simulink model "ofdm_txrx_supermimo"  (Simulink 7.1)
settings: Solver=VariableStepDiscrete  SolverMode=SingleTasking  StartTime=0.0  StopTime=simOnly_simLength  RelTol=1e-3
PostLoadFcn:
  | ofdm_rx_supermimo_init
InitFcn:
  | ofdm_rx_supermimo_init
StartFcn:
  | ofdm_rx_supermimo_init
values from neighbouring files (symbols this model uses):
  Action0_Config = 0* 31*2^18 + 0*2^24 + PHY_AUTORESPONSE_REQ_GOODHDR + PHY_AUTORESPONSE_REQ_GOODPKT + PHY_AUTORESPONSE_REQ_FLAGA    [ofdm_tx_supermimo_init.m]
  Action1_Config = 0* 62*2^18 + PHY_AUTORESPONSE_REQ_GOODHDR + PHY_AUTORESPONSE_REQ_GOODPKT    [ofdm_tx_supermimo_init.m]
  Action2_Config = 0    [ofdm_tx_supermimo_init.m]
  Action3_Config = 0    [ofdm_tx_supermimo_init.m]
  Action4_Config = 0    [ofdm_tx_supermimo_init.m]
  Action5_Config = 0    [ofdm_tx_supermimo_init.m]
  ActionID_AFTransmit = 31    [ofdm_tx_supermimo_init.m]
  ActionID_Disabled = 0    [ofdm_tx_supermimo_init.m]
  ActionID_SetFlagA = 62    [ofdm_tx_supermimo_init.m]
  ActionID_SetFlagB = 61    [ofdm_tx_supermimo_init.m]
  BER_RAM_init_size = 2048    [ofdm_tx_supermimo_init.m]
  CPLength = 16    [ofdm_tx_supermimo_init.m]
  longCorr_coef_i = [-4*real(LTS) 0]    [ofdm_rx_supermimo_init.m]
  longCorr_coef_q = [4*imag(LTS) 0]    [ofdm_rx_supermimo_init.m]
  Match0_Config = 0    [ofdm_tx_supermimo_init.m]
  Match1_Config = 0    [ofdm_tx_supermimo_init.m]
  Match2_Config = 0    [ofdm_tx_supermimo_init.m]
  Match3_Config = 0    [ofdm_tx_supermimo_init.m]
  Match4_Config = 0    [ofdm_tx_supermimo_init.m]
  Match5_Config = 0    [ofdm_tx_supermimo_init.m]
  Match6_Config = 0    [ofdm_tx_supermimo_init.m]
  Match7_Config = 0    [ofdm_tx_supermimo_init.m]
  max_CP_length = 16    [ofdm_tx_supermimo_init.m]
  max_num_baseRateSymbols = 31    [ofdm_tx_supermimo_init.m]
  max_num_subcarriers = 64    [ofdm_tx_supermimo_init.m]
  max_num_trainingSymbols = 15    [ofdm_tx_supermimo_init.m]
  max_numBytes = 16384    [ofdm_tx_supermimo_init.m]
  max_OFDM_symbols = 511    [ofdm_tx_supermimo_init.m]
  modConstellation_qam16 = .75*[1 3 -1 -3]./3    [ofdm_tx_supermimo_init.m]
  modConstellation_qam64 = 0.875*[3 1 5 7 -3 -1 -5 -7]./7    [ofdm_tx_supermimo_init.m]
  modConstellation_qpsk = [1 -1]./sqrt(2)    [ofdm_tx_supermimo_init.m]
  numBaseRateSymbols = ceil(numHeaderBytes / numBytes_BaseRateOFDMSymbol)    [ofdm_tx_supermimo_init.m]
  numSubcarriers = 64    [ofdm_tx_supermimo_init.m]
  numTrainingSymbols = 2    [ofdm_tx_supermimo_init.m]
  pilot_indicies = 7 + ( (64-21) * 2^8) + ( (64-7) * 2^16) + (21 * 2^24)    (= 356068103)    [ofdm_tx_supermimo_init.m]
  pilotValues = hex2dec('A57D') + (2^16 * hex2dec('5A82'))    [ofdm_tx_supermimo_init.m]
  pkt_crossCorr_thresh = 0.7    [ofdm_rx_supermimo_init.m]
  pkt_energy_thresh = 0    [ofdm_rx_supermimo_init.m]
  pktByteNums = numHeaderBytes +  				byteIndex_numPayloadBytes(1)*2^8 +  				byteIndex_numPayloadBytes(2)*2^16 +  				byteIndex_simpleDynModMasks * 2^24    [ofdm_rx_supermimo_init.m]
  PktDet_Delay = 58+32 +      Symbol_Timing_Offset*2^7 +      0*2^16 +      (117* 2^24)    (= 1962935642)    [ofdm_rx_supermimo_init.m]
  pktTiming_count_payloadStart = pktTiming_count_lsStart + pktTiming_ls_num*pktTiming_ls_length    [ofdm_rx_supermimo_init.m]
  preamble = preamble_scale*[shortsyms_10 longsyms_2]    [ofdm_tx_supermimo_init.m]
  preamble_ROM = preamble_I_ROM + preamble_Q_ROM*2^16    [ofdm_tx_supermimo_init.m]
  RAM_init_size = max_numBytes    (= 16384)    [ofdm_tx_supermimo_init.m]
  reg_AF_Tx_Blanking = (320+80) + ( (320+80+79)*2^16)    (= 31392144)    [ofdm_rx_supermimo_init.m]
  reg_AF_Tx_Scaling = 2944    [ofdm_rx_supermimo_init.m]
  reg_Interrupt_PktBuf_Control = reg_InterruptControl +  	1 * 2^16 +  	0 * 2^24    (= 65656)    [ofdm_rx_supermimo_init.m]
  reg_PilotCalcParams = (4 * 2^16) + (minPilotChanMag)    (= 262184.96)    [ofdm_rx_supermimo_init.m]
  regRx_chanEst_minMag = ((0.5)*2^15) + ((0.5 * 2^15)*2^16)    (= 1073758208)    [ofdm_rx_supermimo_init.m]
  regRx_coarseCalcCorrection = round((1.3e-3 - 1.2982e-3)*2^32)    [ofdm_rx_supermimo_init.m]
  regRx_pilotCalcCorrection = round(1.00115 * 2^31)    [ofdm_rx_supermimo_init.m]
  regRx_preCFOoptions = 0 * 2^0 +  	1 * 2^1 +  	0    (= 2)    [ofdm_rx_supermimo_init.m]
  rx_controlBits = 1 * 2  		 + 1 * 4  		 + 0 * 8  		 + 1 * 16 * rx_SISO_Mode  		 + 1 * 32  		 + 1 * 64  		 + 1 * 128  		 + 1 * 256  		 + 1 * 512  		 + 1 * 1024  		 + 1 * 4096  		 + 0 * 16384  		 + 0 * 32768  		 + 1 * 65536  		 + 0 * 131072  		 + 0 * 262144  		 + 1 * 524288  		 + 1 * 1048576 * tx_Alamouti_Mode  		 + 0 * 2097152           + 1 * 33554432  		 + 0 * 4194304  		 + 0 * 8388608  		 + 0 * 16777216  		 + 1 *  ...(+49 chars)    [ofdm_rx_supermimo_init.m]
  Rx_PktDet_LongCorr_Params = LongCorrCounterLoad + 0*2^8 + 0*2^16 + 255*2^24    (= 4278190325)    [ofdm_rx_supermimo_init.m]
  Rx_PktDet_LongCorrThresholds = 8000    [ofdm_rx_supermimo_init.m]
  rx_postEq_scaling = round(rxScaling*2^11) * (1 + 2^16)    [ofdm_rx_supermimo_init.m]
  RxDataScrambling_Seq = [40 198 78 63 82 173 102 245 48 111 172 115 147 230 216 93 72 65 62 2 205 242 122 90 128 83 105 97 73 10 5 252]    [ofdm_tx_supermimo_init.m]
  RxReg_FixedPktLen = 0    [ofdm_rx_supermimo_init.m]
  shortSymbol_time = shortSymbol_time(1:16).'    [ofdm_tx_supermimo_init.m]
  simOnly_numSamples = length(preamble)+( (numSubcarriers+CPLength)*(numTrainingSymbols + numBaseRateSymbols + numFullRateSymbols/2) )    [ofdm_tx_supermimo_init.m]
  train = [train train]    [ofdm_tx_supermimo_init.m]
  tx_controlBits = (antB_preambleShift * 2^4) + (2^1 * tx_Alamouti_Mode) +  	(2^0 * tx_SISO_Mode) + 4*0 + 8*1 + 0*256 + 512*0 + 1024*0 +  	0*hex2dec('1000') + 0*hex2dec('2000') + 0*hex2dec('4000') +  	1*hex2dec('8000') + 1*hex2dec('10000') + hex2dec('20000') +  	16*hex2dec('100000')    [ofdm_tx_supermimo_init.m]
  tx_delays = (50*2^0) + (0*2^8) + (30*2^12)    (= 122930)    [ofdm_tx_supermimo_init.m]
  tx_scaling = (3072) + (12288 * 2^16)    (= 805309440)    [ofdm_tx_supermimo_init.m]
  TxDataScrambling_Seq = [40 198 78 63 82 173 102 245 48 111 172 115 147 230 216 93 72 65 62 2 205 242 122 90 128 83 105 97 73 10 5 252]    [ofdm_tx_supermimo_init.m]
  txfilt_firpm = 0.95*txfilt_firpm./(max(txfilt_firpm))    [ofdm_tx_supermimo_init.m]
  txfilt_intfilt = [0 intfilt(4,4,.8)]    [ofdm_tx_supermimo_init.m]
  txReg_symbolCounts = (2^8 *numBaseRateSymbols ) + numTrainingSymbols    [ofdm_tx_supermimo_init.m]
  TxRx_FFTScaling = (3 * 2^0) +  	(2 * 2^2) +  	(1 * 2^4) +  	(1 * 2^6) +  	(1 * 2^8) +  	(0 * 2^10)    (= 347)    [ofdm_tx_supermimo_init.m]
undefined symbols (not defined in the file or in its folder): AntA, AntB, BaseRate, Down, gcbh, numBuffs, numBytes, Up, xlBlockLoadCallback, xlGetSimulinkPeriod, xlipgetpartsetting
libraries: xbsIndex_r4 x6035, simulink x4, dspsigops x4, commchan3 x2, dspsrcs4 x2, commrflib2 x2
(part 25 of 61)

== system ofdm_txrx_supermimo/OFDM Rx MIMO/Packet_Detection/Internal PktDet (Sim Only!)/Magnitude Squared ==
  I : Inport  OutDataType=sfix(16)  OutScaling=2^0
  Q : Inport  Port=2  OutDataType=sfix(16)  OutScaling=2^0
  AddSub1 : xbsIndex_r4/AddSub  mode=Addition  use_carryin=off  use_carryout=off  en=off  latency=addLatency  precision=User Defined  arith_type=Unsigned  n_bits=prec  bin_pt=bp  quantization=Truncate  overflow=Saturate  dbl_ovrd=off  use_behavioral_HDL=on  pipelined=off  use_rpm=on  hw_selection=Fabric  block_type=addsub
      settings: xl_area=[12 22 0 22 0 0 0]  block_version=VER_STRING_GOES_HERE
  Mult1 : xbsIndex_r4/Mult  precision=User Defined  arith_type="Signed  (2's comp)"  n_bits=prec  bin_pt=bp+1  quantization=Truncate  overflow=Saturate  en=off  latency=multLatency  dbl_ovrd=off  use_behavioral_HDL=off  use_embedded=on  opt=Speed  optimum_pipeline=off  pipeline=on  use_rpm=off  placement_style=Rectangular shape  block_type=mult
      settings: infoedit=Hardware notes: To use the internal pipeline stage of the dedicated multiplier you must select 'Pipeline for maximum performance'.  xl_area=[63 105 0 106 0 4 0]  block_version=VER_STRING_GOES_HERE
  Mult4 : xbsIndex_r4/Mult  precision=User Defined  arith_type="Signed  (2's comp)"  n_bits=prec  bin_pt=bp+1  quantization=Truncate  overflow=Saturate  en=off  latency=multLatency  dbl_ovrd=off  use_behavioral_HDL=off  use_embedded=on  opt=Speed  optimum_pipeline=off  pipeline=on  use_rpm=off  placement_style=Rectangular shape  block_type=mult
      settings: infoedit=Hardware notes: To use the internal pipeline stage of the dedicated multiplier you must select 'Pipeline for maximum performance'.  xl_area=[63 105 0 106 0 4 0]  block_version=VER_STRING_GOES_HERE
  Mag : Outport  OutDataType=sfix(16)  OutScaling=2^0
  wires:
  AddSub1/1 -> Mag/1
  Mult1/1 -> AddSub1/2
  Mult4/1 -> AddSub1/1
  Q/1 -> Mult1/2, Mult1/1
  I/1 -> Mult4/2, Mult4/1

== system ofdm_txrx_supermimo/OFDM Rx MIMO/Packet_Detection/Internal PktDet (Sim Only!)/Magnitude Squared1 ==
  I : Inport  OutDataType=sfix(16)  OutScaling=2^0
  Q : Inport  Port=2  OutDataType=sfix(16)  OutScaling=2^0
  AddSub1 : xbsIndex_r4/AddSub  mode=Addition  use_carryin=off  use_carryout=off  en=off  latency=addLatency  precision=User Defined  arith_type=Unsigned  n_bits=prec  bin_pt=bp  quantization=Truncate  overflow=Saturate  dbl_ovrd=off  use_behavioral_HDL=on  pipelined=off  use_rpm=on  hw_selection=Fabric  block_type=addsub
      settings: xl_area=[9 0 0 17 0 0 0]  block_version=VER_STRING_GOES_HERE
  Mult1 : xbsIndex_r4/Mult  precision=User Defined  arith_type="Signed  (2's comp)"  n_bits=prec  bin_pt=bp+1  quantization=Truncate  overflow=Saturate  en=off  latency=multLatency  dbl_ovrd=off  use_behavioral_HDL=off  use_embedded=on  opt=Speed  optimum_pipeline=off  pipeline=on  use_rpm=off  placement_style=Rectangular shape  block_type=mult
      settings: infoedit=Hardware notes: To use the internal pipeline stage of the dedicated multiplier you must select 'Pipeline for maximum performance'.  xl_area=[9 17 0 17 0 1 0]  block_version=VER_STRING_GOES_HERE
  Mult4 : xbsIndex_r4/Mult  precision=User Defined  arith_type="Signed  (2's comp)"  n_bits=prec  bin_pt=bp+1  quantization=Truncate  overflow=Saturate  en=off  latency=multLatency  dbl_ovrd=off  use_behavioral_HDL=off  use_embedded=on  opt=Speed  optimum_pipeline=off  pipeline=on  use_rpm=off  placement_style=Rectangular shape  block_type=mult
      settings: infoedit=Hardware notes: To use the internal pipeline stage of the dedicated multiplier you must select 'Pipeline for maximum performance'.  xl_area=[9 17 0 17 0 1 0]  block_version=VER_STRING_GOES_HERE
  Mag : Outport  OutDataType=sfix(16)  OutScaling=2^0
  wires:
  I/1 -> Mult4/1, Mult4/2
  Q/1 -> Mult1/1, Mult1/2
  Mult4/1 -> AddSub1/1
  Mult1/1 -> AddSub1/2
  AddSub1/1 -> Mag/1

== system ofdm_txrx_supermimo/OFDM Rx MIMO/Packet_Detection/Internal PktDet (Sim Only!)/Sliding Window ==
  In : Inport  OutDataType=sfix(16)  OutScaling=2^0
  Accum1 : xbsIndex_r4/Accumulator  operation=Add  n_bits=22  overflow=Flag as error  scale=1  rst=off  hasbypass=off  en=off  dbl_ovrd=off  use_behavioral_HDL=on  implementation=Fabric  block_type=accum
      settings: infoedit="Adder or subtractor-based accumulator.   Output type and binary point position match the input.<P><P>Hardware notes: When \"Reinitialize with input 'b' on reset\" is selected, the accumulator is forced to run at the system rate even if the input 'b' is running at a slower rate."  xl_area=[12 23 0 22 0 0 0]  block_version=VER_STRING_GOES_HERE
  AddSub1 : xbsIndex_r4/AddSub  mode=Subtraction  use_carryin=off  use_carryout=off  en=off  latency=1  precision=Full  arith_type="Signed  (2's comp)"  n_bits=36  bin_pt=28  quantization=Truncate  overflow=Saturate  dbl_ovrd=off  use_behavioral_HDL=on  pipelined=off  use_rpm=off  hw_selection=Fabric  block_type=addsub
      settings: xl_area=[9 18 0 17 0 0 0]  block_version=VER_STRING_GOES_HERE
  Delay6 : xbsIndex_r4/Delay  en=off  latency=16  dbl_ovrd=off  reg_retiming=off  block_type=delay
      settings: infoedit=Hardware notes: A delay line is a chain, each link of which is an SRL16 followed by a flip-flop. If register retiming is enabled, the delay line is a chain of flip-flops.  xl_area=[9 17 0 17 0 0 0]  block_version=VER_STRING_GOES_HERE
  Out : Outport  OutDataType=sfix(16)  OutScaling=2^0
  wires:
  Accum1/1 -> Out/1
  In/1 -> Delay6/1, AddSub1/1
  AddSub1/1 -> Accum1/1
  Delay6/1 -> AddSub1/2

== system ofdm_txrx_supermimo/OFDM Rx MIMO/Packet_Detection/Internal PktDet (Sim Only!)/Sliding Window1 ==
  In : Inport  OutDataType=sfix(16)  OutScaling=2^0
  Accum1 : xbsIndex_r4/Accumulator  operation=Add  n_bits=22  overflow=Flag as error  scale=1  rst=off  hasbypass=off  en=off  dbl_ovrd=off  use_behavioral_HDL=on  implementation=Fabric  block_type=accum
      settings: infoedit="Adder or subtractor-based accumulator.   Output type and binary point position match the input.<P><P>Hardware notes: When \"Reinitialize with input 'b' on reset\" is selected, the accumulator is forced to run at the system rate even if the input 'b' is running at a slower rate."  xl_area=[12 23 0 22 0 0 0]  block_version=VER_STRING_GOES_HERE
  AddSub1 : xbsIndex_r4/AddSub  mode=Subtraction  use_carryin=off  use_carryout=off  en=off  latency=1  precision=Full  arith_type="Signed  (2's comp)"  n_bits=36  bin_pt=28  quantization=Truncate  overflow=Saturate  dbl_ovrd=off  use_behavioral_HDL=on  pipelined=off  use_rpm=off  hw_selection=Fabric  block_type=addsub
      settings: xl_area=[9 18 0 17 0 0 0]  block_version=VER_STRING_GOES_HERE
  Delay6 : xbsIndex_r4/Delay  en=off  latency=16  dbl_ovrd=off  reg_retiming=off  block_type=delay
      settings: infoedit=Hardware notes: A delay line is a chain, each link of which is an SRL16 followed by a flip-flop. If register retiming is enabled, the delay line is a chain of flip-flops.  xl_area=[9 17 0 17 0 0 0]  block_version=VER_STRING_GOES_HERE
  Out : Outport  OutDataType=sfix(16)  OutScaling=2^0
  wires:
  Delay6/1 -> AddSub1/2
  AddSub1/1 -> Accum1/1
  In/1 -> AddSub1/1, Delay6/1
  Accum1/1 -> Out/1

== system ofdm_txrx_supermimo/OFDM Rx MIMO/Packet_Detection/Internal PktDet (Sim Only!)/Sliding Window2 ==
  In : Inport  OutDataType=sfix(16)  OutScaling=2^0
  Accum1 : xbsIndex_r4/Accumulator  operation=Add  n_bits=22  overflow=Flag as error  scale=1  rst=off  hasbypass=off  en=off  dbl_ovrd=off  use_behavioral_HDL=on  implementation=Fabric  block_type=accum
      settings: infoedit="Adder or subtractor-based accumulator.   Output type and binary point position match the input.<P><P>Hardware notes: When \"Reinitialize with input 'b' on reset\" is selected, the accumulator is forced to run at the system rate even if the input 'b' is running at a slower rate."  xl_area=[12 23 0 22 0 0 0]  block_version=VER_STRING_GOES_HERE
  AddSub1 : xbsIndex_r4/AddSub  mode=Subtraction  use_carryin=off  use_carryout=off  en=off  latency=0  precision=Full  arith_type="Signed  (2's comp)"  n_bits=36  bin_pt=28  quantization=Truncate  overflow=Saturate  dbl_ovrd=off  use_behavioral_HDL=on  pipelined=off  use_rpm=off  hw_selection=Fabric  block_type=addsub
      settings: xl_area=[9 0 0 17 0 0 0]  block_version=VER_STRING_GOES_HERE
  Delay6 : xbsIndex_r4/Delay  en=off  latency=16  dbl_ovrd=off  reg_retiming=off  block_type=delay
      settings: infoedit=Hardware notes: A delay line is a chain, each link of which is an SRL16 followed by a flip-flop. If register retiming is enabled, the delay line is a chain of flip-flops.  xl_area=[9 17 0 17 0 0 0]  block_version=VER_STRING_GOES_HERE
  Out : Outport  OutDataType=sfix(16)  OutScaling=2^0
  wires:
  Accum1/1 -> Out/1
  In/1 -> Delay6/1, AddSub1/1
  AddSub1/1 -> Accum1/1
  Delay6/1 -> AddSub1/2

== system ofdm_txrx_supermimo/OFDM Rx MIMO/Packet_Detection/RSSI-based PktDet/Ant A Carrier Sensing ==
  Avg RSSI : Inport  OutDataType=sfix(16)  OutScaling=2^0
  Convert : xbsIndex_r4/Convert  arith_type=Unsigned  n_bits=1  bin_pt=0  quantization=Truncate  overflow=Wrap  latency=0  dbl_ovrd=off  pipeline=off  block_type=convert
      settings: block_version=8.2
  Convert1 : xbsIndex_r4/Convert  arith_type=Unsigned  n_bits=1  bin_pt=0  quantization=Truncate  overflow=Wrap  latency=0  dbl_ovrd=off  pipeline=off  block_type=convert
      settings: block_version=8.2
  Inverter : xbsIndex_r4/Inverter  en=off  latency=0  dbl_ovrd=off  block_type=inv
  Inverter1 : xbsIndex_r4/Inverter  en=off  latency=0  dbl_ovrd=off  block_type=inv
      settings: block_version=8.2
  Logical6 : xbsIndex_r4/Logical  logical_function=AND  inputs=2  en=off  latency=0  precision=Full  arith_type=Unsigned  n_bits=16  bin_pt=0  align_bp=on  dbl_ovrd=off  block_type=logical
      settings: block_version=8.2
  Logical7 : xbsIndex_r4/Logical  logical_function=OR  inputs=2  en=off  latency=0  precision=Full  arith_type=Unsigned  n_bits=16  bin_pt=0  align_bp=on  dbl_ovrd=off  block_type=logical
      settings: block_version=8.2
  Relational1 : xbsIndex_r4/Relational  mode=a>b  en=off  latency=0  dbl_ovrd=off  block_type=relational
      settings: block_version=VER_STRING_GOES_HERE
  busy : Outport  OutDataType=sfix(16)  OutScaling=2^0
  idle : Outport  Port=2  OutDataType=sfix(16)  OutScaling=2^0
  wires:
  Avg RSSI/1 -> Relational1/1
  Relational1/1 -> Convert/1
  Convert/1 -> Inverter/1, Logical6/2
  [regPktDet_carrierSenseThresh] -> Relational1/2
  Inverter/1 -> Logical7/1
  [regPktDet_pktDetMask_A] -> Convert1/1
  Inverter1/1 -> Logical7/2
  Logical6/1 -> busy/1
  Logical7/1 -> idle/1
  Convert1/1 -> Inverter1/1, Logical6/1

== system ofdm_txrx_supermimo/OFDM Rx MIMO/Packet_Detection/RSSI-based PktDet/Ant B Carrier Sensing ==
  Avg RSSI : Inport  OutDataType=sfix(16)  OutScaling=2^0
  Convert1 : xbsIndex_r4/Convert  arith_type=Unsigned  n_bits=1  bin_pt=0  quantization=Truncate  overflow=Wrap  latency=0  dbl_ovrd=off  pipeline=off  block_type=convert
      settings: block_version=8.2
  Convert2 : xbsIndex_r4/Convert  arith_type=Unsigned  n_bits=1  bin_pt=0  quantization=Truncate  overflow=Wrap  latency=0  dbl_ovrd=off  pipeline=off  block_type=convert
      settings: block_version=8.2
  Inverter : xbsIndex_r4/Inverter  en=off  latency=0  dbl_ovrd=off  block_type=inv
  Inverter1 : xbsIndex_r4/Inverter  en=off  latency=0  dbl_ovrd=off  block_type=inv
      settings: block_version=8.2
  Logical6 : xbsIndex_r4/Logical  logical_function=AND  inputs=2  en=off  latency=0  precision=Full  arith_type=Unsigned  n_bits=16  bin_pt=0  align_bp=on  dbl_ovrd=off  block_type=logical
      settings: block_version=8.2
  Logical7 : xbsIndex_r4/Logical  logical_function=OR  inputs=2  en=off  latency=0  precision=Full  arith_type=Unsigned  n_bits=16  bin_pt=0  align_bp=on  dbl_ovrd=off  block_type=logical
      settings: block_version=8.2
  Relational1 : xbsIndex_r4/Relational  mode=a>b  en=off  latency=0  dbl_ovrd=off  block_type=relational
      settings: block_version=VER_STRING_GOES_HERE
  busy : Outport  OutDataType=sfix(16)  OutScaling=2^0
  idle : Outport  Port=2  OutDataType=sfix(16)  OutScaling=2^0
  wires:
  Logical7/1 -> idle/1
  Logical6/1 -> busy/1
  Inverter1/1 -> Logical7/2
  [regPktDet_pktDetMask_B] -> Convert2/1
  Inverter/1 -> Logical7/1
  [regPktDet_carrierSenseThresh] -> Relational1/2
  Convert1/1 -> Logical6/2, Inverter/1
  Relational1/1 -> Convert1/1
  Avg RSSI/1 -> Relational1/1
  Convert2/1 -> Logical6/1, Inverter1/1

== system ofdm_txrx_supermimo/OFDM Rx MIMO/Packet_Detection/RSSI-based PktDet/AntA Detector ==
  RSSI : Inport  OutDataType=sfix(16)  OutScaling=2^0
  Reset : Inport  Port=2  OutDataType=sfix(16)  OutScaling=2^0
  Detection Decision : Subsystem
  Gateway Out : xbsIndex_r4/Gateway Out  hdl_port=off  timing_constraint=None  locs_specified=off  LOCs={}  block_type=gatewayout
      settings: infoedit="Gateway out block.  Converts Xilinx fixed point inputs into ouputs of type Simulink integer, double, or fixed point.<P><P>Hardware notes:  In hardware these blocks become top level output ports or are discarded, depending on how they are configured."  block_version=8.2
  Gateway Out1 : xbsIndex_r4/Gateway Out  hdl_port=off  timing_constraint=None  locs_specified=off  LOCs={}  block_type=gatewayout
      settings: infoedit="Gateway out block.  Converts Xilinx fixed point inputs into ouputs of type Simulink integer, double, or fixed point.<P><P>Hardware notes:  In hardware these blocks become top level output ports or are discarded, depending on how they are configured."  block_version=8.2
  Gateway Out2 : xbsIndex_r4/Gateway Out  hdl_port=off  timing_constraint=None  locs_specified=off  LOCs={}  block_type=gatewayout
      settings: infoedit="Gateway out block.  Converts Xilinx fixed point inputs into ouputs of type Simulink integer, double, or fixed point.<P><P>Hardware notes:  In hardware these blocks become top level output ports or are discarded, depending on how they are configured."  block_version=8.2
  Pkt Det Decision : Scope  NumInputPorts=3
      settings: YMin=-1~-1~-1  YMax=1~1~1
  RF Gain Adj : Subsystem
  Running Sum : Subsystem
  pktdet : Outport  OutDataType=sfix(16)  OutScaling=2^0
  avgRSSI : Outport  Port=2  OutDataType=sfix(16)  OutScaling=2^0
  tag scope: regPktDet_pktDetected_AntA=global
  wires:
  Running Sum/1(avg) -> avgRSSI/1, Gateway Out1/1, Detection Decision/1(Avg RSSI)
  [regPktDet_MasterReset] -> Running Sum/2(reset)
  [regPktDet_RSSIavgLength] -> Running Sum/3(avglen)
  RF Gain Adj/1(" RSSI") -> Running Sum/1(RSSI), Gateway Out/1
  Gateway Out/1 -> Pkt Det Decision/1  "RAWRSSI"
  Gateway Out1/1 -> Pkt Det Decision/2  "AVGRSSI"
  Gateway Out2/1 -> Pkt Det Decision/3  "PKTDET"
  Reset/1 -> Detection Decision/2(Reset)
  Detection Decision/1(Pkt Det) -> pktdet/1, Gateway Out2/1, [regPktDet_pktDetected_AntA]
  RSSI/1 -> RF Gain Adj/1(RSSI)

== system ofdm_txrx_supermimo/OFDM Rx MIMO/Packet_Detection/RSSI-based PktDet/AntB Detector ==
  RSSI : Inport  OutDataType=sfix(16)  OutScaling=2^0
  Reset : Inport  Port=2  OutDataType=sfix(16)  OutScaling=2^0
  Detection Decision : Subsystem
  Gateway Out : xbsIndex_r4/Gateway Out  hdl_port=off  timing_constraint=None  locs_specified=off  LOCs={}  block_type=gatewayout
      settings: infoedit="Gateway out block.  Converts Xilinx fixed point inputs into ouputs of type Simulink integer, double, or fixed point.<P><P>Hardware notes:  In hardware these blocks become top level output ports or are discarded, depending on how they are configured."  block_version=8.2
  Gateway Out1 : xbsIndex_r4/Gateway Out  hdl_port=off  timing_constraint=None  locs_specified=off  LOCs={}  block_type=gatewayout
      settings: infoedit="Gateway out block.  Converts Xilinx fixed point inputs into ouputs of type Simulink integer, double, or fixed point.<P><P>Hardware notes:  In hardware these blocks become top level output ports or are discarded, depending on how they are configured."  block_version=8.2
  Gateway Out2 : xbsIndex_r4/Gateway Out  hdl_port=off  timing_constraint=None  locs_specified=off  LOCs={}  block_type=gatewayout
      settings: infoedit="Gateway out block.  Converts Xilinx fixed point inputs into ouputs of type Simulink integer, double, or fixed point.<P><P>Hardware notes:  In hardware these blocks become top level output ports or are discarded, depending on how they are configured."  block_version=8.2
  Pkt Det Decision : Scope  NumInputPorts=3
      settings: YMin=-1~-1~-1  YMax=1~1~1
  RF Gain Adj : Subsystem
  Running Sum : Subsystem
  pktdet : Outport  OutDataType=sfix(16)  OutScaling=2^0
  avgRSSI : Outport  Port=2  OutDataType=sfix(16)  OutScaling=2^0
  tag scope: regPktDet_pktDetected_AntB=global
  wires:
  Detection Decision/1(Pkt Det) -> Gateway Out2/1, pktdet/1, [regPktDet_pktDetected_AntB]
  Reset/1 -> Detection Decision/2(Reset)
  Gateway Out2/1 -> Pkt Det Decision/3  "PKTDET"
  Gateway Out1/1 -> Pkt Det Decision/2  "AVGRSSI"
  Gateway Out/1 -> Pkt Det Decision/1  "RAWRSSI"
  RF Gain Adj/1(" RSSI") -> Gateway Out/1, Running Sum/1(RSSI)
  [regPktDet_RSSIavgLength] -> Running Sum/3(avglen)
  [regPktDet_MasterReset] -> Running Sum/2(reset)
  Running Sum/1(avg) -> Gateway Out1/1, avgRSSI/1, Detection Decision/1(Avg RSSI)
  RSSI/1 -> RF Gain Adj/1(RSSI)

== system ofdm_txrx_supermimo/OFDM Rx MIMO/Packet_Detection/RSSI-based PktDet/DIFS Counter ==
  Busy : Inport
  Idle : Inport  Port=2
  Convert1 : xbsIndex_r4/Convert  arith_type=Unsigned  n_bits=1  bin_pt=0  quantization=Truncate  overflow=Wrap  latency=0  dbl_ovrd=off  pipeline=off  block_type=convert
      settings: block_version=VER_STRING_GOES_HERE
  Convert2 : xbsIndex_r4/Convert  arith_type=Boolean  n_bits=1  bin_pt=0  quantization=Truncate  overflow=Wrap  latency=0  dbl_ovrd=off  pipeline=off  block_type=convert
      settings: block_version=VER_STRING_GOES_HERE
  Convert3 : xbsIndex_r4/Convert  arith_type=Boolean  n_bits=1  bin_pt=0  quantization=Truncate  overflow=Wrap  latency=0  dbl_ovrd=off  pipeline=off  block_type=convert
      settings: block_version=VER_STRING_GOES_HERE
  Counter1 : xbsIndex_r4/Counter  cnt_type=Free Running  cnt_to=Inf  operation=Up  start_count=0  cnt_by_val=1  arith_type=Unsigned  n_bits=10  bin_pt=0  load_pin=off  rst=on  en=on  explicit_period=off  period=4  dbl_ovrd=off  use_behavioral_HDL=off  use_rpm=off  implementation=Fabric  block_type=counter
  Inverter1 : xbsIndex_r4/Inverter  en=off  latency=0  dbl_ovrd=off  block_type=inv
      settings: xl_area=[0 0 0 0 0 0 0]  block_version=8.2
  Logical1 : xbsIndex_r4/Logical  logical_function=OR  inputs=2  en=off  latency=0  precision=Full  arith_type=Unsigned  n_bits=16  bin_pt=0  align_bp=on  dbl_ovrd=off  block_type=logical
  Relational3 : xbsIndex_r4/Relational  mode=a>b  en=off  latency=0  dbl_ovrd=off  block_type=relational
      settings: block_version=VER_STRING_GOES_HERE
  S-R Latch : Subsystem
  Idle for DIFS : Outport
  tag scope: regPktDet_idleCounter=global
  wires:
  Convert1/1 -> Idle for DIFS/1
  Idle/1 -> Convert2/1
  S-R Latch/1(Q) -> Convert1/1
  Relational3/1 -> S-R Latch/2(S)
  [regPktDet_DIFSperiod] -> Relational3/2
  Counter1/1 -> Relational3/1, [regPktDet_idleCounter]
  Inverter1/1 -> Logical1/2
  Logical1/1 -> Counter1/1
  Busy/1 -> Convert3/1
  Convert2/1 -> Counter1/2, Inverter1/1
  Convert3/1 -> S-R Latch/1(R), Logical1/1

== system ofdm_txrx_supermimo/OFDM Rx MIMO/Packet_Detection/RSSI-based PktDet/Ext PktDet ==
  Constant2 : Constant  Value=0  OutDataType=sfix(16)  OutScaling=2^0
  Convert5 : xbsIndex_r4/Convert  arith_type=Boolean  n_bits=1  bin_pt=0  quantization=Truncate  overflow=Flag as error  latency=0  dbl_ovrd=off  pipeline=off  block_type=convert
      settings: block_version=10.1.3
  Down Sample1 : xbsIndex_r4/Down Sample  sample_ratio=4  sample_phase="Last Value of Frame  (most efficient)"  en=off  latency=1  dbl_ovrd=off  block_type=dsamp
      settings: block_version=VER_STRING_GOES_HERE
  Ext_PktDet : xbsIndex_r4/Gateway In  arith_type=Unsigned  n_bits=1  bin_pt=0  quantization="Round  (unbiased: +/- Inf)"  overflow=Saturate  period=1  dbl_ovrd=off  timing_constraint=None  locs_specified=off  LOCs={}  block_type=gatewayin
      settings: infoedit="Gateway in block.  Converts inputs of type Simulink integer, double and fixed point to  Xilinx fixed point type.<P><P>Hardware notes:  In hardware these blocks become top level input ports."  block_version=VER_STRING_GOES_HERE
  Logical2 : xbsIndex_r4/Logical  logical_function=AND  inputs=2  en=off  latency=0  precision=Full  arith_type=Unsigned  n_bits=16  bin_pt=0  align_bp=on  dbl_ovrd=off  block_type=logical
      settings: block_version=8.2
  Det : Outport
  wires:
  Ext_PktDet/1 -> Down Sample1/1
  Constant2/1 -> Ext_PktDet/1
  [regPktDet_EnableExtDet] -> Logical2/2
  Logical2/1 -> Det/1
  Convert5/1 -> Logical2/1
  Down Sample1/1 -> Convert5/1

== system ofdm_txrx_supermimo/OFDM Rx MIMO/Packet_Detection/RSSI-based PktDet/MIMO Logic ==
  Det A : Inport  OutDataType=sfix(16)  OutScaling=2^0
  Det B : Inport  Port=2  OutDataType=sfix(16)  OutScaling=2^0
  Convert{1..4,7} (5 alike) : xbsIndex_r4/Convert  arith_type=Unsigned  n_bits=1  bin_pt=0  quantization=Truncate  overflow=Flag as error  latency=0  dbl_ovrd=off  pipeline=off  block_type=convert
      settings: block_version=10.1.3
  Logical : xbsIndex_r4/Logical  logical_function=AND  inputs=2  en=off  latency=0  precision=Full  arith_type=Unsigned  n_bits=16  bin_pt=0  align_bp=on  dbl_ovrd=off  block_type=logical
  Logical1 : xbsIndex_r4/Logical  logical_function=AND  inputs=2  en=off  latency=0  precision=Full  arith_type=Unsigned  n_bits=16  bin_pt=0  align_bp=on  dbl_ovrd=off  block_type=logical
  Logical2 : xbsIndex_r4/Logical  logical_function=OR  inputs=2  en=off  latency=0  precision=Full  arith_type=Unsigned  n_bits=16  bin_pt=0  align_bp=on  dbl_ovrd=off  block_type=logical
  Logical3 : xbsIndex_r4/Logical  logical_function=AND  inputs=2  en=off  latency=0  precision=Full  arith_type=Unsigned  n_bits=16  bin_pt=0  align_bp=on  dbl_ovrd=off  block_type=logical
  Mux : xbsIndex_r4/Mux  inputs=2  en=off  latency=0  precision=Full  arith_type=Unsigned  n_bits=16  bin_pt=14  quantization=Truncate  overflow=Wrap  dbl_ovrd=off  block_type=mux
  PktDet : Outport  OutDataType=sfix(16)  OutScaling=2^0
  wires:
  [regPktDet_pktDetMode] -> Convert4/1
  [regPktDet_pktDetMask_A] -> Convert2/1
  [regPktDet_pktDetMask_B] -> Convert3/1
  Det A/1 -> Convert7/1
  Det B/1 -> Convert1/1
  Convert7/1 -> Logical/1
  Convert2/1 -> Logical/2
  Convert1/1 -> Logical1/1
  Convert3/1 -> Logical1/2
  Logical/1 -> Logical2/1, Logical3/1
  Logical1/1 -> Logical2/2, Logical3/2
  Logical2/1 -> Mux/3
  Convert4/1 -> Mux/1
  Logical3/1 -> Mux/2
  Mux/1 -> PktDet/1

== system ofdm_txrx_supermimo/OFDM Rx MIMO/Packet_Detection/RSSI-based PktDet/Mid-Packet RSSI Register ==
  AntB : Inport
  AntA : Inport  Port=2
  Payload : Inport  Port=3  OutDataType=sfix(16)  OutScaling=2^0
  Concat1 : xbsIndex_r4/Concat  num_inputs=2  dbl_ovrd=off  block_type=concat
      settings: block_version=10.1.3
  Convert4 : xbsIndex_r4/Convert  arith_type=Boolean  n_bits=1  bin_pt=0  quantization=Truncate  overflow=Wrap  latency=0  dbl_ovrd=off  pipeline=off  block_type=convert
      settings: block_version=10.1.3
  Convert5 : xbsIndex_r4/Convert  arith_type=Unsigned  n_bits=16  bin_pt=0  quantization=Truncate  overflow=Wrap  latency=0  dbl_ovrd=off  pipeline=off  block_type=convert
      settings: block_version=10.1.3
  Convert6 : xbsIndex_r4/Convert  arith_type=Unsigned  n_bits=16  bin_pt=0  quantization=Truncate  overflow=Wrap  latency=0  dbl_ovrd=off  pipeline=off  block_type=convert
      settings: block_version=10.1.3
  Terminator : Terminator
  To Register2 : xbsIndex_r4/To Register  shared_memory_name='midPacketRSSI'  init=0  ownership=Locally owned and initialized  explicit_data_type=on  arith_type=Unsigned  n_bits=32  bin_pt=0  dbl_ovrd=off  block_type=toreg
      settings: AttributesFormatString=<< %<shared_memory_name> >>  infoedit="Register block that writes data to a shared memory register.  Delay of one sample period."  block_version=10.1.3
  posedge1 : Subsystem
  wires:
  Payload/1 -> posedge1/1(In)
  posedge1/1(Out) -> Convert4/1
  Concat1/1 -> To Register2/1
  To Register2/1 -> Terminator/1
  Convert4/1 -> To Register2/2
  Convert5/1 -> Concat1/1
  Convert6/1 -> Concat1/2
  AntB/1 -> Convert5/1
  AntA/1 -> Convert6/1

== system ofdm_txrx_supermimo/OFDM Rx MIMO/Packet_Detection/RSSI-based PktDet/Posedge ==
  D : Inport  OutDataType=sfix(16)  OutScaling=2^0
  Delay : xbsIndex_r4/Delay  en=off  latency=1  dbl_ovrd=off  reg_retiming=off  block_type=delay
      settings: infoedit=Hardware notes: A delay line is a chain, each link of which is an SRL16 followed by a flip-flop. If register retiming is enabled, the delay line is a chain of flip-flops.  xl_area=[1 1 0 0 0 0 0]  block_version=VER_STRING_GOES_HERE
  Inverter : xbsIndex_r4/Inverter  en=off  latency=0  dbl_ovrd=off  block_type=inv
      settings: xl_area=[0 0 0 0 0 0 0]  block_version=VER_STRING_GOES_HERE
  Logical : xbsIndex_r4/Logical  logical_function=AND  inputs=2  en=off  latency=0  precision=Full  arith_type=Unsigned  n_bits=8  bin_pt=2  align_bp=on  dbl_ovrd=off  block_type=logical
      settings: xl_area=[1 0 0 1 0 0 0]  block_version=VER_STRING_GOES_HERE
  Q : Outport  OutDataType=sfix(16)  OutScaling=2^0
  wires:
  Logical/1 -> Q/1
  D/1 -> Delay/1, Logical/1
  Inverter/1 -> Logical/2
  Delay/1 -> Inverter/1

== system ofdm_txrx_supermimo/OFDM Rx MIMO/Packet_Detection/RSSI-based PktDet/RSSI Clock Generator ==
  note: The RSSI ADC on the radio board can be clocked up to 20MHz.
      | We clock it at 10MHz (1/4 the I/Q samping rate). This
      | clock is generated here by a 20MHz 2-bit counter. If the LBS is used,
      | the RSSI clock will be CLK/2 (10MHz). If the second LSB is used,
      | it will be CLK/4 (5MHz).
  2-bit Counter : xbsIndex_r4/Counter  cnt_type=Free Running  cnt_to=Inf  operation=Up  start_count=0  cnt_by_val=1  arith_type=Unsigned  n_bits=2  bin_pt=0  load_pin=off  rst=off  en=off  explicit_period=on  period=2  dbl_ovrd=off  use_behavioral_HDL=off  use_rpm=off  implementation=Fabric  block_type=counter
      settings: block_version=VER_STRING_GOES_HERE
  LSB : xbsIndex_r4/Slice  nbits=1  boolean_output=off  mode=Lower Bit Location + Width  bit1=0  base1=MSB of Input  bit0=0  base0=LSB of Input  dbl_ovrd=off  block_type=slice
      settings: infoedit="Extracts a given range of bits from each input sample and presents it at the output.  The output type is ordinarily unsigned with binary point at zero, but can be Boolean when the slice is one bit wide.<P><P>Hardware notes: In hardware this block costs nothing."  block_version=8.2.02
  LSB1 : xbsIndex_r4/Slice  nbits=1  boolean_output=off  mode=Lower Bit Location + Width  bit1=0  base1=MSB of Input  bit0=1  base0=LSB of Input  dbl_ovrd=off  block_type=slice
      settings: infoedit="Extracts a given range of bits from each input sample and presents it at the output.  The output type is ordinarily unsigned with binary point at zero, but can be Boolean when the slice is one bit wide.<P><P>Hardware notes: In hardware this block costs nothing."  block_version=8.2.02
  Mux : xbsIndex_r4/Mux  inputs=2  en=off  latency=0  precision=Full  arith_type=Unsigned  n_bits=16  bin_pt=14  quantization=Truncate  overflow=Wrap  dbl_ovrd=off  block_type=mux
      settings: block_version=8.2.02
  RSSI Clock : Scope
      settings: SaveName=ScopeData3
  RSSI_CLK_OUT : xbsIndex_r4/Gateway Out  hdl_port=on  timing_constraint=None  locs_specified=off  LOCs={}  block_type=gatewayout
      settings: infoedit="Gateway out block.  Converts Xilinx fixed point inputs into ouputs of type Simulink integer, double, or fixed point.<P><P>Hardware notes:  In hardware these blocks become top level output ports or are discarded, depending on how they are configured."  block_version=VER_STRING_GOES_HERE
  wires:
  [regPktDet_RSSIclkRatioSel] -> Mux/1
  LSB1/1 -> Mux/3
  LSB/1 -> Mux/2
  Mux/1 -> RSSI_CLK_OUT/1
  RSSI_CLK_OUT/1 -> RSSI Clock/1
  2-bit Counter/1 -> LSB/1, LSB1/1

== system ofdm_txrx_supermimo/OFDM Rx MIMO/Packet_Timing_Control/Disable for Interrupts/S-R Latch ==
  S : Inport  OutDataType=sfix(16)  OutScaling=2^0
  R : Inport  Port=2  OutDataType=sfix(16)  OutScaling=2^0
  Constant1 : xbsIndex_r4/Constant  arith_type=Boolean  const=1  n_bits=1  bin_pt=0  explicit_period=off  period=1  equ=P=C  opselect=C  inp2=PCIN>>17  opr=+  inp1=P  carry=CIN  dbl_ovrd=off  block_type=constant
      settings: block_version=VER_STRING_GOES_HERE
  Register2 : xbsIndex_r4/Register  init=0  rst=on  en=on  dbl_ovrd=off  block_type=register
      settings: xl_area=[1 1 0 0 0 0 0]  block_version=VER_STRING_GOES_HERE
  Q : Outport  OutDataType=sfix(16)  OutScaling=2^0
  wires:
  Constant1/1 -> Register2/1
  R/1 -> Register2/2
  Register2/1 -> Q/1
  S/1 -> Register2/3

== system ofdm_txrx_supermimo/OFDM Rx MIMO/Packet_Timing_Control/Ext Reset/debounce ==
  D : Inport  OutDataType=sfix(16)  OutScaling=2^0
  Delay{,1..3} (4 alike) : xbsIndex_r4/Delay  Orientation=down  en=off  latency=1  dbl_ovrd=off  reg_retiming=off  block_type=delay
      settings: infoedit=Hardware notes: A delay line is a chain, each link of which is an SRL16 followed by a flip-flop. If register retiming is enabled, the delay line is a chain of flip-flops.  xl_area=[1 1 0 0 0 0 0]  block_version=8.2
  Logical : xbsIndex_r4/Logical  logical_function=AND  inputs=5  en=off  latency=0  precision=Full  arith_type=Unsigned  n_bits=16  bin_pt=0  align_bp=on  dbl_ovrd=off  block_type=logical
      settings: xl_area=[1 0 0 1 0 0 0]  block_version=8.2
  Q : Outport  OutDataType=sfix(16)  OutScaling=2^0
  wires:
  Logical/1 -> Q/1
  D/1 -> Delay/1, Logical/1
  Delay/1 -> Delay1/1, Logical/2
  Delay1/1 -> Delay2/1, Logical/3
  Delay2/1 -> Delay3/1, Logical/4
  Delay3/1 -> Logical/5

== system ofdm_txrx_supermimo/OFDM Tx MIMO/OutputBuffers/Addr Gen/1 Shift Reg ==
  d : Inport  OutDataType=sfix(16)  OutScaling=2^0
  rst : Inport  Port=2  OutDataType=sfix(16)  OutScaling=2^0
  en : Inport  Port=3  OutDataType=sfix(16)  OutScaling=2^0
  Payload Running1 : xbsIndex_r4/Register  init=0  rst=on  en=on  dbl_ovrd=off  block_type=register
      settings: xl_area=[1 1 0 0 0 0 0]  block_version=VER_STRING_GOES_HERE
  Q : Outport  OutDataType=sfix(16)  OutScaling=2^0
  wires:
  en/1 -> Payload Running1/3
  rst/1 -> Payload Running1/2
  d/1 -> Payload Running1/1
  Payload Running1/1 -> Q/1

== system ofdm_txrx_supermimo/OFDM Tx MIMO/OutputBuffers/Addr Gen/1 Shift Reg1 ==
  d : Inport  OutDataType=sfix(16)  OutScaling=2^0
  rst : Inport  Port=2  OutDataType=sfix(16)  OutScaling=2^0
  en : Inport  Port=3  OutDataType=sfix(16)  OutScaling=2^0
  Payload Running1 : xbsIndex_r4/Register  init=1  rst=on  en=on  dbl_ovrd=off  block_type=register
      settings: xl_area=[1 1 0 0 0 0 0]  block_version=VER_STRING_GOES_HERE
  Q : Outport  OutDataType=sfix(16)  OutScaling=2^0
  wires:
  Payload Running1/1 -> Q/1
  d/1 -> Payload Running1/1
  rst/1 -> Payload Running1/2
  en/1 -> Payload Running1/3

== system ofdm_txrx_supermimo/OFDM Tx MIMO/OutputBuffers/Addr Gen/2 Shift Reg ==
  d : Inport  OutDataType=sfix(16)  OutScaling=2^0
  en : Inport  Port=2  OutDataType=sfix(16)  OutScaling=2^0
  rst : Inport  Port=3  OutDataType=sfix(16)  OutScaling=2^0
  Payload Running1 : xbsIndex_r4/Register  init=0  rst=on  en=on  dbl_ovrd=off  block_type=register
      settings: xl_area=[1 1 0 0 0 0 0]  block_version=VER_STRING_GOES_HERE
  Payload Running3 : xbsIndex_r4/Register  init=0  rst=on  en=on  dbl_ovrd=off  block_type=register
      settings: xl_area=[1 1 0 0 0 0 0]  block_version=VER_STRING_GOES_HERE
  Q : Outport  OutDataType=sfix(16)  OutScaling=2^0
  wires:
  en/1 -> Payload Running1/3, Payload Running3/3
  rst/1 -> Payload Running1/2, Payload Running3/2
  d/1 -> Payload Running1/1
  Payload Running3/1 -> Q/1
  Payload Running1/1 -> Payload Running3/1

== system ofdm_txrx_supermimo/OFDM Tx MIMO/OutputBuffers/Addr Gen/Oscillator1 ==
  en : Inport  OutDataType=sfix(16)  OutScaling=2^0
  rst : Inport  Port=2  OutDataType=sfix(16)  OutScaling=2^0
  Assert : xbsIndex_r4/Assert  Orientation=left  assert_type=on  type_source=Explicitly  arith_type=Boolean  n_bits=16  bin_pt=14  assert_rate=on  rate_source=Explicitly  period=2  output_port=on  block_type=assert
      settings: infoedit=Asserts a user-defined sample rate and/or type on a signal.<P><P>Hardware notes: In hardware this block costs nothing.  block_version=VER_STRING_GOES_HERE
  Inverter1 : xbsIndex_r4/Inverter  Orientation=left  en=off  latency=0  dbl_ovrd=off  block_type=inv
      settings: block_version=VER_STRING_GOES_HERE
  Register : xbsIndex_r4/Register  init=0  rst=on  en=on  dbl_ovrd=off  block_type=register
      settings: block_version=VER_STRING_GOES_HERE
  Q : Outport  OutDataType=sfix(16)  OutScaling=2^0
  wires:
  Register/1 -> Assert/1, Q/1
  en/1 -> Register/3
  Inverter1/1 -> Register/1
  Assert/1 -> Inverter1/1
  rst/1 -> Register/2

== system ofdm_txrx_supermimo/OFDM Tx MIMO/OutputBuffers/Addr Gen/Read Addr Generator ==
  note: About the addresses:
      | The IFFT output time series [0,1,...63] is written to a RAM with an offset of 16, modulo 64, so that:
      | Xn=[0,1,...63] -> WrAddr=[16,17,...15]
      |
      | This allows much easier read address generation, which must include both the full IFFT output and a cyclic prefix.
      |
      | For SISO/Multiplexing MIMO modes, the output series per symbol must be:
      | Xn_out = [48,49...63,0,1,...63] - 80 samples long ({CP SYM} = {SYM[48:63] SYM[0:63]})
      |
      | Thus, the 80-sample sequence of read addresses is
      | RdAddr=[0,1,...15,16,17,...63,0,1,...15]
      |
      | For Alamouti mode, the non-conjugated symbols are handled the same as the SISO/Multiplexing mode. For the
      | conjugated symbols, we use the Fourier identity of:
      | if x(t) = ifft(X(f)) then
      | ifft(conj(X(f))) = conj(x(-t))
      |
      | So we can "generate" the conjugate of the frequency domain symbol by reading the time domain series (which we
      | already have, since Alamouti uses the same symbol twice) backwards, then conjugating. Notice that the zero'th entry
      | in the time series doesn't change, but the rest do.
      |
      | So, for conjugated Alamouti symbols:
      | Xn_out = [16,15,14,...0,63,...2,1] - 80 saples long {CP SYM} = {SYM[16:1] SYM[0] SYM[63:1]}
      | Thus,
      | RdAddr = [32,31,...0,63,62,...17]
  En : Inport  OutDataType=sfix(16)  OutScaling=2^0
  Rst : Inport  Port=2  OutDataType=sfix(16)  OutScaling=2^0
  SwapDir : Inport  Port=3  OutDataType=sfix(16)  OutScaling=2^0
  6LSB : xbsIndex_r4/Slice  nbits=log2(numSubcarriers)  boolean_output=off  mode=Lower Bit Location + Width  bit1=0  base1=MSB of Input  bit0=0  base0=LSB of Input  dbl_ovrd=off  block_type=slice
      settings: infoedit="Extracts a given range of bits from each input sample and presents it at the output.  The output type is ordinarily unsigned with binary point at zero, but can be Boolean when the slice is one bit wide.<P><P>Hardware notes: In hardware this block costs nothing."  block_version=VER_STRING_GOES_HERE
  6LSB1 : xbsIndex_r4/Slice  nbits=log2(numSubcarriers)  boolean_output=off  mode=Lower Bit Location + Width  bit1=0  base1=MSB of Input  bit0=0  base0=LSB of Input  dbl_ovrd=off  block_type=slice
      settings: infoedit="Extracts a given range of bits from each input sample and presents it at the output.  The output type is ordinarily unsigned with binary point at zero, but can be Boolean when the slice is one bit wide.<P><P>Hardware notes: In hardware this block costs nothing."  block_version=VER_STRING_GOES_HERE
  AddSub : xbsIndex_r4/AddSub  mode=Addition  use_carryin=off  use_carryout=off  en=off  latency=0  precision=Full  arith_type=Unsigned  n_bits=16  bin_pt=14  quantization=Truncate  overflow=Wrap  dbl_ovrd=off  use_behavioral_HDL=off  pipelined=off  use_rpm=on  hw_selection=Fabric  block_type=addsub
      settings: block_version=8.2.02
  Constant1 : xbsIndex_r4/Constant  arith_type=Unsigned  const=32  n_bits=ceil(log2(numSubcarriers + CPLength - 1))  bin_pt=0  explicit_period=off  period=2  equ=P=C  opselect=C  inp2=PCIN>>17  opr=+  inp1=P  carry=CIN  dbl_ovrd=off  block_type=constant
      settings: block_version=VER_STRING_GOES_HERE
  Constant3 : xbsIndex_r4/Constant  Orientation=left  arith_type=Unsigned  const=numSubcarriers + CPLength - 1  n_bits=ceil(log2(numSubcarriers + CPLength - 1))  bin_pt=0  explicit_period=off  period=2  equ=P=C  opselect=C  inp2=PCIN>>17  opr=+  inp1=P  carry=CIN  dbl_ovrd=off  block_type=constant
      settings: block_version=VER_STRING_GOES_HERE
  Down Sample2 : xbsIndex_r4/Down Sample  sample_ratio=2  sample_phase=First Value of Frame  en=off  latency=0  dbl_ovrd=off  block_type=dsamp
      settings: block_version=VER_STRING_GOES_HERE
  Logical : xbsIndex_r4/Logical  Orientation=up  logical_function=OR  inputs=2  en=off  latency=0  precision=Full  arith_type=Unsigned  n_bits=16  bin_pt=0  align_bp=on  dbl_ovrd=off  block_type=logical
      settings: block_version=VER_STRING_GOES_HERE
  Mux : xbsIndex_r4/Mux  inputs=2  en=off  latency=0  precision=Full  arith_type=Unsigned  n_bits=16  bin_pt=14  quantization=Truncate  overflow=Wrap  dbl_ovrd=off  block_type=mux
      settings: block_version=8.2.02
  Negate : xbsIndex_r4/Negate  precision=Full  arith_type="Signed  (2's comp)"  n_bits=16  bin_pt=14  quantization=Truncate  overflow=Wrap  en=off  latency=0  dbl_ovrd=off  block_type=negate
      settings: block_version=8.2.02
  Read Counter : xbsIndex_r4/Counter  cnt_type=Free Running  cnt_to=numSubcarriers + CPLength - 1  operation=Up  start_count=0  cnt_by_val=1  arith_type=Unsigned  n_bits=ceil(log2(numSubcarriers + CPLength - 1))  bin_pt=0  load_pin=off  rst=on  en=on  explicit_period=off  period=2  dbl_ovrd=off  use_behavioral_HDL=off  use_rpm=off  implementation=Fabric  block_type=counter
      settings: block_version=VER_STRING_GOES_HERE
  Relational : xbsIndex_r4/Relational  Orientation=left  mode=a=b  en=off  latency=0  dbl_ovrd=off  block_type=relational
      settings: block_version=VER_STRING_GOES_HERE
  Up Sample : xbsIndex_r4/Up Sample  sample_ratio=2  copy_samples=off  dbl_ovrd=off  block_type=usamp
      settings: infoedit="Up samples input data.  Inserted values  can be zeros or copies of the most recent input sample.<P><P>Hardware notes: No hardware is needed if inserted values are copies of the input sample; otherwise, a mux and single bit flip-flop are used."  block_version=VER_STRING_GOES_HERE
  Q : Outport  OutDataType=sfix(16)  OutScaling=2^0
  Ovfl : Outport  Port=2  OutDataType=sfix(16)  OutScaling=2^0
  Slot : Outport  Port=3  OutDataType=sfix(16)  OutScaling=2^0
  wires:
  Read Counter/1 -> Relational/1, AddSub/1, 6LSB/1
  Relational/1 -> Logical/2, Up Sample/1
  Logical/1 -> Read Counter/1
  Constant3/1 -> Relational/2
  En/1 -> Read Counter/2
  Rst/1 -> Logical/1
  Up Sample/1 -> Ovfl/1
  AddSub/1 -> Negate/1
  Negate/1 -> 6LSB1/1
  Constant1/1 -> AddSub/2
  Mux/1 -> Q/1
  6LSB/1 -> Mux/2
  6LSB1/1 -> Mux/3
  SwapDir/1 -> Down Sample2/1
  Down Sample2/1 -> Mux/1, Slot/1

== system ofdm_txrx_supermimo/OFDM Tx MIMO/OutputBuffers/Addr Gen/SR Latch ==
  S : Inport  OutDataType=sfix(16)  OutScaling=2^0
  R : Inport  Port=2  OutDataType=sfix(16)  OutScaling=2^0
  Constant1 : xbsIndex_r4/Constant  arith_type=Boolean  const=1  n_bits=1  bin_pt=0  explicit_period=off  period=2  equ=P=C  opselect=C  inp2=PCIN>>17  opr=+  inp1=P  carry=CIN  dbl_ovrd=off  block_type=constant
      settings: block_version=VER_STRING_GOES_HERE
  Payload Running3 : xbsIndex_r4/Register  init=0  rst=on  en=on  dbl_ovrd=off  block_type=register
      settings: xl_area=[1 1 0 0 0 0 0]  block_version=VER_STRING_GOES_HERE
  Q : Outport  OutDataType=sfix(16)  OutScaling=2^0
  wires:
  Constant1/1 -> Payload Running3/1
  R/1 -> Payload Running3/2
  S/1 -> Payload Running3/3
  Payload Running3/1 -> Q/1

== system ofdm_txrx_supermimo/OFDM Tx MIMO/OutputBuffers/Addr Gen/Subsystem ==
  note: Alamouti mode treats base rate and full rate symbols
      | the same, so the memory address generation logic
      | ignore BaseRate for Alamouti transmissions.
  BaseRate : Inport
  Rst : Inport  Port=2
  En : Inport  Port=3
  Training : Inport  Port=4
  Mux : xbsIndex_r4/Mux  inputs=2  en=off  latency=0  precision=Full  arith_type=Unsigned  n_bits=16  bin_pt=14  quantization=Truncate  overflow=Wrap  dbl_ovrd=off  block_type=mux
  Oscillator : Subsystem
  Oscillator2 : Subsystem
  Q : Outport
  wires:
  Oscillator2/1(Q) -> Mux/2  "Wr Bank Sel"
  Oscillator/1(Q) -> Mux/3
  BaseRate/1 -> Oscillator2/1(period)
  Rst/1 -> Oscillator2/2(rst), Oscillator/1(rst)
  En/1 -> Oscillator2/3(en), Oscillator/2(en)
  Training/1 -> Oscillator/3(Period)
  Mux/1 -> Q/1
  [TxReg_Alamouti_Mode] -> Mux/1

== system ofdm_txrx_supermimo/OFDM Tx MIMO/OutputBuffers/RAMs/Alamouti Encoding ==
  note: In Alamouti mode, even and odd syms are sent via
      | both antennas, in anternating order. In MIMO mode,
      | even/odd syms are mapped to antennas A/B.
  Slot : Inport  OutDataType=sfix(16)  OutScaling=2^0
  EvenSyms_I : Inport  Port=2  OutDataType=sfix(16)  OutScaling=2^0
  EvenSyms_Q : Inport  Port=3  OutDataType=sfix(16)  OutScaling=2^0
  OddSyms_I : Inport  Port=4  OutDataType=sfix(16)  OutScaling=2^0
  OddSyms_Q : Inport  Port=5  OutDataType=sfix(16)  OutScaling=2^0
  Mux{,1..3} (4 alike) : xbsIndex_r4/Mux  inputs=2  en=off  latency=0  precision=Full  arith_type=Unsigned  n_bits=16  bin_pt=14  quantization=Truncate  overflow=Wrap  dbl_ovrd=off  block_type=mux
      settings: block_version=8.2.02
  Negate : xbsIndex_r4/Negate  precision=User Defined  arith_type="Signed  (2's comp)"  n_bits=14  bin_pt=13  quantization=Truncate  overflow=Saturate  en=off  latency=0  dbl_ovrd=off  block_type=negate
      settings: block_version=8.2.02
  Negate1 : xbsIndex_r4/Negate  precision=User Defined  arith_type="Signed  (2's comp)"  n_bits=14  bin_pt=13  quantization=Truncate  overflow=Saturate  en=off  latency=0  dbl_ovrd=off  block_type=negate
      settings: block_version=8.2.02
  AntA_I : Outport  OutDataType=sfix(16)  OutScaling=2^0
  AntA_Q : Outport  Port=2  OutDataType=sfix(16)  OutScaling=2^0
  AntB_I : Outport  Port=3  OutDataType=sfix(16)  OutScaling=2^0
  AntB_Q : Outport  Port=4  OutDataType=sfix(16)  OutScaling=2^0
  wires:
  Mux/1 -> AntB_Q/1
  Mux3/1 -> AntA_I/1
  Mux2/1 -> AntA_Q/1
  Mux1/1 -> AntB_I/1
  Slot/1 -> Mux1/1, Mux/1, Mux2/1, Mux3/1
  EvenSyms_I/1 -> Mux3/2, Mux1/3
  EvenSyms_Q/1 -> Mux2/2, Negate1/1
  OddSyms_I/1 -> Mux1/2, Negate/1
  OddSyms_Q/1 -> Mux/2, Mux2/3
  Negate/1 -> Mux3/3
  Negate1/1 -> Mux/3
